simulink model "Simulatore_COMAU_UBHAND_IV_manipolazione_interna"  (Simulink 7.6)
settings: Solver=ode45  StartTime=0.0  StopTime=inf  RelTol=1e-3
values from neighbouring files (symbols this model uses):
  a_f = zeros(4,5)    [KinematicData.m]
  alpha_f = zeros(4,5)    [KinematicData.m]
  d_f = zeros(4,5)    [KinematicData.m]
  dc4_1 = 660    [KinematicData.m]
  T6HAND = [1 0 0 0;0 1 0 0; 0 0 1 357.48+95; 0 0 0 1]    [KinematicData.m]
  teta_d = atan(647.07/130)    [KinematicData.m]
  teta_f = zeros(4,5)    [KinematicData.m]
undefined symbols (not defined in the file or in its folder): a_c, a_w, alpha_c, alpha_w, d_c, d_w, Dt_fingers, teta_c
libraries: simulink x281, dspmtrx3 x171, aerolibutil x163, aerolibconvert2 x64, simulink_extras x46, vrlib x26, aerolibtransform2 x7, dspnetwork x1
(part 9 of 15)

== system Simulatore_COMAU_UBHAND_IV_manipolazione_interna/Work Space Control/Inverse_kinematic/Inverse_kinematic_comau/first solution/q1 ==
  P06 : Inport
  Divide : Product  Inputs=*/
  Gain : Gain  Gain=-1
  P06(1) : dspmtrx3/Submatrix  RowSpan=One row  RowStartMode=Index  RowStartIndex=1  RowEndMode=Index  RowEndIndex=3  ColSpan=One column  ColStartMode=Index  ColStartIndex=1  ColEndMode=Offset from last  ColEndIndex=1
  P06(2) : dspmtrx3/Submatrix  RowSpan=One row  RowStartMode=Index  RowStartIndex=2  RowEndMode=Index  RowEndIndex=3  ColSpan=One column  ColStartMode=Index  ColStartIndex=1  ColEndMode=Offset from last  ColEndIndex=1
  Trigonometric Function : Trigonometry  Operator=atan
  q1 : Outport
  wires:
  P06/1 -> P06(2)/1, P06(1)/1
  Trigonometric Function/1 -> Gain/1
  ? -> ?
  P06(2)/1 -> Divide/1
  P06(1)/1 -> Divide/2
  Divide/1 -> Trigonometric Function/1
  Gain/1 -> q1/1

== system Simulatore_COMAU_UBHAND_IV_manipolazione_interna/Work Space Control/Inverse_kinematic/Inverse_kinematic_comau/first solution/q2 ==
  P06 : Inport
  q1 : Inport  Port=2
  e_quadro : Inport  Port=3
  Add1 : Sum  Inputs=+-
  Add2 : Sum  Inputs=++-
  Add3 : Sum  Inputs=+-
  Add4 : Sum  Inputs=+-
  Angle Conversion1 : aerolibconvert2/Angle Conversion  IU=rad  OU=deg
  Angle Conversion2 : aerolibconvert2/Angle Conversion  IU=rad  OU=deg
  Constant1 : Constant  Value=2
  Constant2 : Constant  Value=a_c(2)
  Constant3 : Constant  Value=dc4_1
  Constant4 : Constant  Value=450
  Constant7 : Constant  Value=a_c(1)
  Display : Display
  Display1 : Display
  Display2 : Display
  Divide4 : Product  Inputs=***
  Divide5 : Product  Inputs=*/
  Math Function1 : Math  Operator=sqrt  SaturateOnIntegerOverflow=on
  Math Function5 : Math  Operator=square  SaturateOnIntegerOverflow=on
  Math Function6 : Math  Operator=square  SaturateOnIntegerOverflow=on
  P06(1) : dspmtrx3/Submatrix  RowSpan=One row  RowStartMode=Index  RowStartIndex=1  RowEndMode=Index  RowEndIndex=3  ColSpan=One column  ColStartMode=Index  ColStartIndex=1  ColEndMode=Offset from last  ColEndIndex=1
  P06(3) : dspmtrx3/Submatrix  RowSpan=One row  RowStartMode=Index  RowStartIndex=3  RowEndMode=Index  RowEndIndex=3  ColSpan=One column  ColStartMode=Index  ColStartIndex=1  ColEndMode=Offset from last  ColEndIndex=1
  Trigonometric Function2 : Trigonometry  Operator=cos
  Trigonometric Function3 : Trigonometry  Operator=acos
  cos_teta : Product  Inputs=*/
  fi : Trigonometry  Operator=atan2
  q2 : Outport
  wires:
  P06/1 -> P06(1)/1, P06(3)/1
  fi/1 -> Add3/1, Angle Conversion1/1
  Trigonometric Function3/1 -> Add3/2, Angle Conversion2/1
  Add3/1 -> q2/1
  q1/1 -> Trigonometric Function2/1
  P06(1)/1 -> Divide5/1
  Trigonometric Function2/1 -> Divide5/2
  Divide5/1 -> Add4/1
  Constant7/1 -> Add4/2
  Angle Conversion1/1 -> Display/1
  Angle Conversion2/1 -> Display1/1
  P06(3)/1 -> Add1/1
  Constant4/1 -> Add1/2
  Constant2/1 -> Divide4/2, Math Function5/1
  Constant3/1 -> Math Function6/1
  Constant1/1 -> Divide4/1
  e_quadro/1 -> Add2/1, Math Function1/1
  Math Function5/1 -> Add2/2
  Math Function6/1 -> Add2/3
  Math Function1/1 -> Divide4/3
  Divide4/1 -> cos_teta/2
  Add2/1 -> cos_teta/1
  cos_teta/1 -> Trigonometric Function3/1, Display2/1
  Add4/1 -> fi/1
  Add1/1 -> fi/2

== system Simulatore_COMAU_UBHAND_IV_manipolazione_interna/Work Space Control/Inverse_kinematic/Inverse_kinematic_hand/Inverse_kinematic_little/Direct_kinematic_index ==
  Joints_reference : Inport  PortDimensions=3
  Constant : Constant  Value=[alpha_f(5,1) alpha_f(5,2) alpha_f(5,3) alpha_f(5,4)]
  Constant2 : Constant  Value=[a_f(5,1) a_f(5,2) a_f(5,3) a_f(5,4)]
  Degrees to Radians : simulink_extras/Transformations/Degrees to Radians
  Demux : Demux
  Demux1 : Demux
  Demux2 : Demux
  Demux3 : Demux  Outputs=3
  Display3 : Display
  Gain : Gain  Gain=0.7
  Matrix Multiply : Product  Multiplication=Matrix(*)
  Matrix Multiply1 : Product  Multiplication=Matrix(*)
  Matrix Multiply2 : Product  Multiplication=Matrix(*)
  Subsystem : Subsystem
  Subsystem1 : Subsystem
  Subsystem2 : Subsystem
  Subsystem3 : Subsystem
  Vector Concatenate : Concatenate  Mode=Vector
  T_finger_tip : Outport
  T_01 : Outport  Port=2
  T_02 : Outport  Port=3
  T_03 : Outport  Port=4
  wires:
  Degrees to Radians/1 -> Demux/1
  Vector Concatenate/1 -> Display3/1, Degrees to Radians/1
  Gain/1 -> Vector Concatenate/2
  Demux3/3 -> Gain/1
  Joints_reference/1 -> Vector Concatenate/1, Demux3/1
  Demux1/4 -> Subsystem3/2(alpha)
  Demux1/3 -> Subsystem2/2(alpha)
  Demux1/2 -> Subsystem1/2(alpha)
  Demux1/1 -> Subsystem/2(alpha)
  Demux2/4 -> Subsystem3/3(a)
  Demux2/3 -> Subsystem2/3(a)
  Demux2/2 -> Subsystem1/3(a)
  Demux2/1 -> Subsystem/3(a)
  Demux/4 -> Subsystem3/1(q)
  Demux/3 -> Subsystem2/1(q)
  Demux/2 -> Subsystem1/1(q)
  Demux/1 -> Subsystem/1(q)
  Constant2/1 -> Demux2/1
  Matrix Multiply2/1 -> T_finger_tip/1, T_03/1
  Matrix Multiply1/1 -> Matrix Multiply2/1
  Subsystem3/1(Out1) -> Matrix Multiply2/2
  Subsystem2/1(Out1) -> Matrix Multiply1/2
  Matrix Multiply/1 -> Matrix Multiply1/1, T_02/1
  Subsystem1/1(Out1) -> Matrix Multiply/2
  Constant/1 -> Demux1/1
  Subsystem/1(Out1) -> Matrix Multiply/1, T_01/1

== system Simulatore_COMAU_UBHAND_IV_manipolazione_interna/Work Space Control/Inverse_kinematic/Inverse_kinematic_hand/Inverse_kinematic_little/jacobian ==
  T_01 : Inport
  T_02 : Inport  Port=2
  T_03 : Inport  Port=3
  T_base : Inport  Port=4
  Display1 : Display
  Matrix Concatenate3 : Concatenate  NumInputs=3  Mode=Multidimensional array  ConcatenateDimension=2
  Matrix Concatenate4 : Concatenate  NumInputs=3  Mode=Multidimensional array  ConcatenateDimension=2
  Matrix Concatenate5 : Concatenate  Mode=Multidimensional array
  z1 : Subsystem
  J : Outport
  wires:
  z1/6(z2xpe-p2) -> Matrix Concatenate4/3
  z1/1(z0) -> Matrix Concatenate3/1
  z1/2(z1) -> Matrix Concatenate3/2
  z1/3(z2) -> Matrix Concatenate3/3
  z1/4(z0xpe-p0) -> Matrix Concatenate4/1
  z1/5(z1xpe-p1) -> Matrix Concatenate4/2
  Matrix Concatenate4/1 -> J/1
  T_01/1 -> z1/1(T_01)
  T_02/1 -> z1/2(T_02)
  T_03/1 -> z1/3(T_03)
  T_base/1 -> z1/4(T_base)

== system Simulatore_COMAU_UBHAND_IV_manipolazione_interna/Work Space Control/Inverse_kinematic/Inverse_kinematic_hand/Inverse_kinematic_middle/Direct_kinematic_index ==
  Joints_reference : Inport  PortDimensions=3
  Constant : Constant  Value=[alpha_f(3,1) alpha_f(3,2) alpha_f(3,3) alpha_f(3,4)]
  Constant2 : Constant  Value=[a_f(3,1) a_f(3,2) a_f(3,3) a_f(3,4)]
  Degrees to Radians : simulink_extras/Transformations/Degrees to Radians
  Demux : Demux
  Demux1 : Demux
  Demux2 : Demux
  Demux3 : Demux  Outputs=3
  Display3 : Display
  Gain : Gain  Gain=0.7
  Matrix Multiply : Product  Multiplication=Matrix(*)
  Matrix Multiply1 : Product  Multiplication=Matrix(*)
  Matrix Multiply2 : Product  Multiplication=Matrix(*)
  Subsystem : Subsystem
  Subsystem1 : Subsystem
  Subsystem2 : Subsystem
  Subsystem3 : Subsystem
  Vector Concatenate : Concatenate  Mode=Vector
  T_finger_tip : Outport
  T_01 : Outport  Port=2
  T_02 : Outport  Port=3
  T_03 : Outport  Port=4
  wires:
  Degrees to Radians/1 -> Demux/1
  Vector Concatenate/1 -> Display3/1, Degrees to Radians/1
  Gain/1 -> Vector Concatenate/2
  Demux3/3 -> Gain/1
  Joints_reference/1 -> Vector Concatenate/1, Demux3/1
  Demux1/4 -> Subsystem3/2(alpha)
  Demux1/3 -> Subsystem2/2(alpha)
  Demux1/2 -> Subsystem1/2(alpha)
  Demux1/1 -> Subsystem/2(alpha)
  Demux2/4 -> Subsystem3/3(a)
  Demux2/3 -> Subsystem2/3(a)
  Demux2/2 -> Subsystem1/3(a)
  Demux2/1 -> Subsystem/3(a)
  Demux/4 -> Subsystem3/1(q)
  Demux/3 -> Subsystem2/1(q)
  Demux/2 -> Subsystem1/1(q)
  Demux/1 -> Subsystem/1(q)
  Constant2/1 -> Demux2/1
  Matrix Multiply2/1 -> T_finger_tip/1, T_03/1
  Matrix Multiply1/1 -> Matrix Multiply2/1
  Subsystem3/1(Out1) -> Matrix Multiply2/2
  Subsystem2/1(Out1) -> Matrix Multiply1/2
  Matrix Multiply/1 -> Matrix Multiply1/1, T_02/1
  Subsystem1/1(Out1) -> Matrix Multiply/2
  Constant/1 -> Demux1/1
  Subsystem/1(Out1) -> Matrix Multiply/1, T_01/1

== system Simulatore_COMAU_UBHAND_IV_manipolazione_interna/Work Space Control/Inverse_kinematic/Inverse_kinematic_hand/Inverse_kinematic_middle/jacobian ==
  T_01 : Inport
  T_02 : Inport  Port=2
  T_03 : Inport  Port=3
  T_base : Inport  Port=4
  Display1 : Display
  Matrix Concatenate3 : Concatenate  NumInputs=3  Mode=Multidimensional array  ConcatenateDimension=2
  Matrix Concatenate4 : Concatenate  NumInputs=3  Mode=Multidimensional array  ConcatenateDimension=2
  Matrix Concatenate5 : Concatenate  Mode=Multidimensional array
  z1 : Subsystem
  J : Outport
  wires:
  T_01/1 -> z1/1(T_01)
  T_02/1 -> z1/2(T_02)
  T_03/1 -> z1/3(T_03)
  z1/6(z2xpe-p2) -> Matrix Concatenate4/3
  z1/1(z0) -> Matrix Concatenate3/1
  z1/2(z1) -> Matrix Concatenate3/2
  z1/3(z2) -> Matrix Concatenate3/3
  z1/4(z0xpe-p0) -> Matrix Concatenate4/1
  z1/5(z1xpe-p1) -> Matrix Concatenate4/2
  T_base/1 -> z1/4(T_base)
  Matrix Concatenate4/1 -> J/1

== system Simulatore_COMAU_UBHAND_IV_manipolazione_interna/Work Space Control/Inverse_kinematic/Inverse_kinematic_hand/Inverse_kinematic_middle1/Direct_kinematic_index ==
  Joints_reference : Inport  PortDimensions=3
  Constant : Constant  Value=[alpha_f(2,1) alpha_f(2,2) alpha_f(2,3) alpha_f(2,4)]
  Constant2 : Constant  Value=[a_f(2,1) a_f(2,2) a_f(2,3) a_f(2,4)]
  Degrees to Radians : simulink_extras/Transformations/Degrees to Radians
  Demux : Demux
  Demux1 : Demux
  Demux2 : Demux
  Demux3 : Demux  Outputs=3
  Display3 : Display
  Gain : Gain  Gain=0.7
  Matrix Multiply : Product  Multiplication=Matrix(*)
  Matrix Multiply1 : Product  Multiplication=Matrix(*)
  Matrix Multiply2 : Product  Multiplication=Matrix(*)
  Subsystem : Subsystem
  Subsystem1 : Subsystem
  Subsystem2 : Subsystem
  Subsystem3 : Subsystem
  Vector Concatenate : Concatenate  Mode=Vector
  T_finger_tip : Outport
  T_01 : Outport  Port=2
  T_02 : Outport  Port=3
  T_03 : Outport  Port=4
  wires:
  Degrees to Radians/1 -> Demux/1
  Vector Concatenate/1 -> Display3/1, Degrees to Radians/1
  Gain/1 -> Vector Concatenate/2
  Demux3/3 -> Gain/1
  Joints_reference/1 -> Vector Concatenate/1, Demux3/1
  Demux1/4 -> Subsystem3/2(alpha)
  Demux1/3 -> Subsystem2/2(alpha)
  Demux1/2 -> Subsystem1/2(alpha)
  Demux1/1 -> Subsystem/2(alpha)
  Demux2/4 -> Subsystem3/3(a)
  Demux2/3 -> Subsystem2/3(a)
  Demux2/2 -> Subsystem1/3(a)
  Demux2/1 -> Subsystem/3(a)
  Demux/4 -> Subsystem3/1(q)
  Demux/3 -> Subsystem2/1(q)
  Demux/2 -> Subsystem1/1(q)
  Demux/1 -> Subsystem/1(q)
  Constant2/1 -> Demux2/1
  Matrix Multiply2/1 -> T_finger_tip/1, T_03/1
  Matrix Multiply1/1 -> Matrix Multiply2/1
  Subsystem3/1(Out1) -> Matrix Multiply2/2
  Subsystem2/1(Out1) -> Matrix Multiply1/2
  Matrix Multiply/1 -> Matrix Multiply1/1, T_02/1
  Subsystem1/1(Out1) -> Matrix Multiply/2
  Constant/1 -> Demux1/1
  Subsystem/1(Out1) -> Matrix Multiply/1, T_01/1

== system Simulatore_COMAU_UBHAND_IV_manipolazione_interna/Work Space Control/Inverse_kinematic/Inverse_kinematic_hand/Inverse_kinematic_middle1/jacobian ==
  T_01 : Inport
  T_02 : Inport  Port=2
  T_03 : Inport  Port=3
  T_base : Inport  Port=4
  Matrix Concatenate3 : Concatenate  NumInputs=3  Mode=Multidimensional array  ConcatenateDimension=2
  Matrix Concatenate4 : Concatenate  NumInputs=3  Mode=Multidimensional array  ConcatenateDimension=2
  z1 : Subsystem
  J : Outport
  wires:
  T_01/1 -> z1/1(T_01)
  T_02/1 -> z1/2(T_02)
  T_03/1 -> z1/3(T_03)
  z1/6(z2xpe-p2) -> Matrix Concatenate4/3
  z1/1(z0) -> Matrix Concatenate3/1
  z1/2(z1) -> Matrix Concatenate3/2
  z1/3(z2) -> Matrix Concatenate3/3
  z1/4(z0xpe-p0) -> Matrix Concatenate4/1
  z1/5(z1xpe-p1) -> Matrix Concatenate4/2
  T_base/1 -> z1/4(T_base)
  Matrix Concatenate4/1 -> J/1

== system Simulatore_COMAU_UBHAND_IV_manipolazione_interna/Work Space Control/Inverse_kinematic/Inverse_kinematic_hand/Inverse_kinematic_ring/Direct_kinematic_index ==
  Joints_reference : Inport  PortDimensions=3
  Constant : Constant  Value=[alpha_f(4,1) alpha_f(4,2) alpha_f(4,3) alpha_f(4,4)]
  Constant2 : Constant  Value=[a_f(4,1) a_f(4,2) a_f(4,3) a_f(4,4)]
  Degrees to Radians : simulink_extras/Transformations/Degrees to Radians
  Demux : Demux
  Demux1 : Demux
  Demux2 : Demux
  Demux3 : Demux  Outputs=3
  Display3 : Display
  Gain : Gain  Gain=0.7
  Matrix Multiply : Product  Multiplication=Matrix(*)
  Matrix Multiply1 : Product  Multiplication=Matrix(*)
  Matrix Multiply2 : Product  Multiplication=Matrix(*)
  Subsystem : Subsystem
  Subsystem1 : Subsystem
  Subsystem2 : Subsystem
  Subsystem3 : Subsystem
  Vector Concatenate : Concatenate  Mode=Vector
  T_finger_tip : Outport
  T_01 : Outport  Port=2
  T_02 : Outport  Port=3
  T_03 : Outport  Port=4
  wires:
  Degrees to Radians/1 -> Demux/1
  Vector Concatenate/1 -> Display3/1, Degrees to Radians/1
  Gain/1 -> Vector Concatenate/2
  Demux3/3 -> Gain/1
  Joints_reference/1 -> Vector Concatenate/1, Demux3/1
  Demux1/4 -> Subsystem3/2(alpha)
  Demux1/3 -> Subsystem2/2(alpha)
  Demux1/2 -> Subsystem1/2(alpha)
  Demux1/1 -> Subsystem/2(alpha)
  Demux2/4 -> Subsystem3/3(a)
  Demux2/3 -> Subsystem2/3(a)
  Demux2/2 -> Subsystem1/3(a)
  Demux2/1 -> Subsystem/3(a)
  Demux/4 -> Subsystem3/1(q)
  Demux/3 -> Subsystem2/1(q)
  Demux/2 -> Subsystem1/1(q)
  Demux/1 -> Subsystem/1(q)
  Constant2/1 -> Demux2/1
  Matrix Multiply2/1 -> T_finger_tip/1, T_03/1
  Matrix Multiply1/1 -> Matrix Multiply2/1
  Subsystem3/1(Out1) -> Matrix Multiply2/2
  Subsystem2/1(Out1) -> Matrix Multiply1/2
  Matrix Multiply/1 -> Matrix Multiply1/1, T_02/1
  Subsystem1/1(Out1) -> Matrix Multiply/2
  Constant/1 -> Demux1/1
  Subsystem/1(Out1) -> Matrix Multiply/1, T_01/1

== system Simulatore_COMAU_UBHAND_IV_manipolazione_interna/Work Space Control/Inverse_kinematic/Inverse_kinematic_hand/Inverse_kinematic_ring/jacobian ==
  T_01 : Inport
  T_02 : Inport  Port=2
  T_03 : Inport  Port=3
  T_base : Inport  Port=4
  Display1 : Display
  Matrix Concatenate3 : Concatenate  NumInputs=3  Mode=Multidimensional array  ConcatenateDimension=2
  Matrix Concatenate4 : Concatenate  NumInputs=3  Mode=Multidimensional array  ConcatenateDimension=2
  Matrix Concatenate5 : Concatenate  Mode=Multidimensional array
  z1 : Subsystem
  J : Outport
  wires:
  T_base/1 -> z1/4(T_base)
  z1/6(z2xpe-p2) -> Matrix Concatenate4/3
  z1/1(z0) -> Matrix Concatenate3/1
  z1/2(z1) -> Matrix Concatenate3/2
  z1/3(z2) -> Matrix Concatenate3/3
  z1/4(z0xpe-p0) -> Matrix Concatenate4/1
  z1/5(z1xpe-p1) -> Matrix Concatenate4/2
  Matrix Concatenate4/1 -> J/1
  T_03/1 -> z1/3(T_03)
  T_02/1 -> z1/2(T_02)
  T_01/1 -> z1/1(T_01)

== system Simulatore_COMAU_UBHAND_IV_manipolazione_interna/Work Space Control/Inverse_kinematic/Inverse_kinematic_hand/Inverse_kinematic_thumb/Direct_kinematic_thumb ==
  Joints_reference : Inport  PortDimensions=3
  Add{,1..4} (5 alike) : Sum
  Constant : Constant  Value=[alpha_f(1,1) alpha_f(1,2) alpha_f(1,3) alpha_f(1,4)]
  Constant1 : Constant  Value=teta_f(1,1)
  Constant2 : Constant  Value=[a_f(1,1) a_f(1,2) a_f(1,3) a_f(1,4)]
  Constant3 : Constant  Value=teta_f(1,2)
  Constant4 : Constant  Value=teta_f(1,3)
  Constant5 : Constant  Value=teta_f(1,4)
  Constant6 : Constant  Value=-20
  Degrees to Radians : simulink_extras/Transformations/Degrees to Radians
  Demux : Demux
  Demux1 : Demux
  Demux2 : Demux
  Demux3 : Demux  Outputs=3
  Display3 : Display
  Gain : Gain  Gain=0.8
  Matrix Multiply : Product  Multiplication=Matrix(*)
  Matrix Multiply1 : Product  Multiplication=Matrix(*)
  Matrix Multiply2 : Product  Multiplication=Matrix(*)
  Subsystem : Subsystem
  Subsystem1 : Subsystem
  Subsystem2 : Subsystem
  Subsystem3 : Subsystem
  Vector Concatenate : Concatenate  Mode=Vector
  T_finger_tip : Outport
  T_01 : Outport  Port=2
  T_02 : Outport  Port=3
  T_03 : Outport  Port=4
  wires:
  Constant/1 -> Demux1/1
  Subsystem1/1(Out1) -> Matrix Multiply/2
  Matrix Multiply/1 -> Matrix Multiply1/1, T_02/1
  Subsystem2/1(Out1) -> Matrix Multiply1/2
  Subsystem3/1(Out1) -> Matrix Multiply2/2
  Matrix Multiply1/1 -> Matrix Multiply2/1
  Matrix Multiply2/1 -> T_finger_tip/1, T_03/1
  Constant2/1 -> Demux2/1
  Demux2/1 -> Subsystem/3(a)
  Demux2/2 -> Subsystem1/3(a)
  Demux2/3 -> Subsystem2/3(a)
  Demux2/4 -> Subsystem3/3(a)
  Demux1/1 -> Subsystem/2(alpha)
  Demux1/2 -> Subsystem1/2(alpha)
  Demux1/3 -> Subsystem2/2(alpha)
  Demux1/4 -> Subsystem3/2(alpha)
  Joints_reference/1 -> Demux3/1, Vector Concatenate/1
  Demux3/3 -> Gain/1
  Vector Concatenate/1 -> Display3/1, Degrees to Radians/1
  Degrees to Radians/1 -> Demux/1
  Constant1/1 -> Add/2
  Demux/1 -> Add/1
  Demux/2 -> Add1/1
  Demux/3 -> Add2/1
  Demux/4 -> Add3/1
  Add2/1 -> Subsystem2/1(q)
  Add1/1 -> Subsystem1/1(q)
  Add/1 -> Subsystem/1(q)
  Constant3/1 -> Add1/2
  Constant4/1 -> Add2/2
  Constant5/1 -> Add3/2
  Constant6/1 -> Add4/2
  Gain/1 -> Add4/1
  Add4/1 -> Vector Concatenate/2
  Add3/1 -> Subsystem3/1(q)
  Subsystem/1(Out1) -> Matrix Multiply/1, T_01/1

== system Simulatore_COMAU_UBHAND_IV_manipolazione_interna/Work Space Control/Inverse_kinematic/Inverse_kinematic_hand/Inverse_kinematic_thumb/jacobian ==
  T_01 : Inport
  T_02 : Inport  Port=2
  T_03 : Inport  Port=3
  T_base : Inport  Port=4
  Display1 : Display
  Matrix Concatenate3 : Concatenate  NumInputs=3  Mode=Multidimensional array  ConcatenateDimension=2
  Matrix Concatenate4 : Concatenate  NumInputs=3  Mode=Multidimensional array  ConcatenateDimension=2
  Matrix Concatenate5 : Concatenate  Mode=Multidimensional array
  z1 : Subsystem
  J : Outport
  wires:
  T_01/1 -> z1/1(T_01)
  T_02/1 -> z1/2(T_02)
  T_03/1 -> z1/3(T_03)
  T_base/1 -> z1/4(T_base)
  z1/6(z2xpe-p2) -> Matrix Concatenate4/3
  z1/1(z0) -> Matrix Concatenate3/1
  z1/2(z1) -> Matrix Concatenate3/2
  z1/3(z2) -> Matrix Concatenate3/3
  z1/4(z0xpe-p0) -> Matrix Concatenate4/1
  z1/5(z1xpe-p1) -> Matrix Concatenate4/2
  Matrix Concatenate4/1 -> J/1
  Matrix Concatenate3/1 -> Display1/1

== system Simulatore_COMAU_UBHAND_IV_manipolazione_interna/Work Space Control/Inverse_kinematic/Tf0/T_0_8/Subsystem ==
  q : Inport
  alpha : Inport  Port=2
  a : Inport  Port=3
  A_01 : aerolibutil/Create 3x3 Matrix
  Constant : Constant  Value=0
  Constant1 : Constant  Value=[0 0 0 1]  VectorParams1D=off
  Constant5 : Constant  Value=d_w(1)
  Display : Display
  Gain : Gain  Gain=-1
  Gain1 : Gain  Gain=-1
  Matrix Concatenation : Concatenate  Mode=Multidimensional array  ConcatenateDimension=2
  Matrix Concatenation1 : Concatenate  Mode=Multidimensional array
  Product{,1..5} (6 alike) : Product  RndMeth=Zero
  Trigonometric Function{,1,6..8,11..12} (7 alike) : Trigonometry  Operator=cos
  Trigonometric Function{2..5,9..10,13} (7 alike) : Trigonometry
  Vector Concatenate : Concatenate  NumInputs=3  Mode=Multidimensional array
  Out1 : Outport
  wires:
  Matrix Concatenation1/1 -> Display/1, Out1/1
  Constant1/1 -> Matrix Concatenation1/2
  Matrix Concatenation/1 -> Matrix Concatenation1/1
  Vector Concatenate/1 -> Matrix Concatenation/2
  A_01/1 -> Matrix Concatenation/1
  Constant5/1 -> Vector Concatenate/3
  Product5/1 -> Vector Concatenate/2
  Product4/1 -> Vector Concatenate/1
  Trigonometric Function13/1 -> Product5/2
  Trigonometric Function12/1 -> Product4/2
  a/1 -> Product5/1, Product4/1
  Trigonometric Function11/1 -> A_01/9
  Trigonometric Function10/1 -> A_01/8
  Constant/1 -> A_01/7
  Gain1/1 -> A_01/6
  Product2/1 -> A_01/5
  Trigonometric Function5/1 -> A_01/4
  Product1/1 -> A_01/3
  Gain/1 -> A_01/2
  Trigonometric Function/1 -> A_01/1
  Product/1 -> Gain/1
  Product3/1 -> Gain1/1
  Trigonometric Function9/1 -> Product3/2
  Trigonometric Function8/1 -> Product3/1
  Trigonometric Function7/1 -> Product2/2
  Trigonometric Function6/1 -> Product2/1
  Trigonometric Function4/1 -> Product1/2
  Trigonometric Function3/1 -> Product1/1
  alpha/1 -> Trigonometric Function11/1, Trigonometric Function10/1, Trigonometric Function9/1, Trigonometric Function7/1, Trigonometric Function4/1, Trigonometric Function1/1
  q/1 -> Trigonometric Function13/1, Trigonometric Function12/1, Trigonometric Function8/1, Trigonometric Function6/1, Trigonometric Function5/1, Trigonometric Function3/1, Trigonometric Function2/1, Trigonometric Function/1
  Trigonometric Function1/1 -> Product/2
  Trigonometric Function2/1 -> Product/1

== system Simulatore_COMAU_UBHAND_IV_manipolazione_interna/Work Space Control/Inverse_kinematic/Tf0/T_0_8/Subsystem1 ==
  q : Inport
  alpha : Inport  Port=2
  a : Inport  Port=3
  A_01 : aerolibutil/Create 3x3 Matrix
  Constant : Constant  Value=0
  Constant1 : Constant  Value=[0 0 0 1]  VectorParams1D=off
  Constant5 : Constant  Value=d_w(2)
  Display : Display
  Gain : Gain  Gain=-1
  Gain1 : Gain  Gain=-1
  Matrix Concatenation : Concatenate  Mode=Multidimensional array  ConcatenateDimension=2
  Matrix Concatenation1 : Concatenate  Mode=Multidimensional array
  Product{,1..5} (6 alike) : Product  RndMeth=Zero
  Trigonometric Function{,1,6..8,11..12} (7 alike) : Trigonometry  Operator=cos
  Trigonometric Function{2..5,9..10,13} (7 alike) : Trigonometry
  Vector Concatenate : Concatenate  NumInputs=3  Mode=Multidimensional array
  Out1 : Outport
  wires:
  Trigonometric Function2/1 -> Product/1
  Trigonometric Function1/1 -> Product/2
  q/1 -> Trigonometric Function/1, Trigonometric Function2/1, Trigonometric Function3/1, Trigonometric Function5/1, Trigonometric Function6/1, Trigonometric Function8/1, Trigonometric Function12/1, Trigonometric Function13/1
  alpha/1 -> Trigonometric Function1/1, Trigonometric Function4/1, Trigonometric Function7/1, Trigonometric Function9/1, Trigonometric Function10/1, Trigonometric Function11/1
  Trigonometric Function3/1 -> Product1/1
  Trigonometric Function4/1 -> Product1/2
  Trigonometric Function6/1 -> Product2/1
  Trigonometric Function7/1 -> Product2/2
  Trigonometric Function8/1 -> Product3/1
  Trigonometric Function9/1 -> Product3/2
  Product3/1 -> Gain1/1
  Product/1 -> Gain/1
  Trigonometric Function/1 -> A_01/1
  Gain/1 -> A_01/2
  Product1/1 -> A_01/3
  Trigonometric Function5/1 -> A_01/4
  Product2/1 -> A_01/5
  Gain1/1 -> A_01/6
  Constant/1 -> A_01/7
  Trigonometric Function10/1 -> A_01/8
  Trigonometric Function11/1 -> A_01/9
  a/1 -> Product5/1, Product4/1
  Trigonometric Function12/1 -> Product4/2
  Trigonometric Function13/1 -> Product5/2
  Product4/1 -> Vector Concatenate/1
  Product5/1 -> Vector Concatenate/2
  Constant5/1 -> Vector Concatenate/3
  A_01/1 -> Matrix Concatenation/1
  Vector Concatenate/1 -> Matrix Concatenation/2
  Matrix Concatenation/1 -> Matrix Concatenation1/1
  Constant1/1 -> Matrix Concatenation1/2
  Matrix Concatenation1/1 -> Out1/1, Display/1

== system Simulatore_COMAU_UBHAND_IV_manipolazione_interna/Work Space Control/Inverse_kinematic/Tf0/T_0_8/wrist_reference_ ==
  Angle Conversion : aerolibconvert2/Angle Conversion  IU=deg  OU=rad
  Constant1 : Constant  Value=[0 90 0]  VectorParams1D=off
  Constant2 : Constant  Value=[0 0 0 ]'  VectorParams1D=off
  Constant4 : Constant  Value=[0 0 0 1]  VectorParams1D=off
  Matrix Concatenate : Concatenate  Mode=Multidimensional array  ConcatenateDimension=2
  Matrix Concatenate1 : Concatenate  Mode=Multidimensional array
  Rotation Angles to Direction Cosine Matrix : aerolibtransform2/Rotation Angles to Direction Cosine Matrix  rotationOrder=ZYX
  TH_W : Outport
  wires:
  Matrix Concatenate1/1 -> TH_W/1
  Rotation Angles to Direction Cosine Matrix/1 -> Matrix Concatenate/1
  Constant4/1 -> Matrix Concatenate1/2
  Matrix Concatenate/1 -> Matrix Concatenate1/1
  Angle Conversion/1 -> Rotation Angles to Direction Cosine Matrix/1
  Constant1/1 -> Angle Conversion/1
  Constant2/1 -> Matrix Concatenate/2

== system Simulatore_COMAU_UBHAND_IV_manipolazione_interna/Work Space Control/Grasp Planner/SCOPES/Direct_kinematic2/Direct_kinematic_Comau/T_0_3 ==
  q[1..3] : Inport
  Add : Sum
  Add1 : Sum
  Add2 : Sum  Inputs=-+
  Constant : Constant  Value=[alpha_c(1) alpha_c(2) alpha_c(3) ]
  Constant1 : Constant  Value=teta_c(2)
  Constant2 : Constant  Value=[a_c(1) a_c(2) a_c(3)]
  Constant3 : Constant  Value=teta_c(3)
  Constant4 : Constant  Value=teta_c(1)
  Degrees to Radians : simulink_extras/Transformations/Degrees to Radians
  Demux : Demux  Outputs=3
  Demux1 : Demux  Outputs=3
  Demux2 : Demux  Outputs=3
  Display3 : Display
  Matrix Multiply : Product  Multiplication=Matrix(*)
  Matrix Multiply1 : Product  Multiplication=Matrix(*)
  Subsystem : Subsystem
  Subsystem1 : Subsystem
  Subsystem2 : Subsystem
  T_0_3 : Outport
  wires:
  Constant/1 -> Demux1/1
  Subsystem/1(Out1) -> Matrix Multiply/1
  Subsystem1/1(Out1) -> Matrix Multiply/2
  Matrix Multiply/1 -> Matrix Multiply1/1
  Subsystem2/1(Out1) -> Matrix Multiply1/2
  Matrix Multiply1/1 -> T_0_3/1
  Constant2/1 -> Demux2/1
  Demux2/1 -> Subsystem/3(a)
  Demux2/2 -> Subsystem1/3(a)
  Demux2/3 -> Subsystem2/3(a)
  Demux1/1 -> Subsystem/2(alpha)
  Demux1/2 -> Subsystem1/2(alpha)
  Demux1/3 -> Subsystem2/2(alpha)
  Degrees to Radians/1 -> Demux/1
  q[1..3]/1 -> Degrees to Radians/1, Display3/1
  Add/1 -> Subsystem1/1(q)
  Constant1/1 -> Add/2
  Demux/2 -> Add/1
  Constant3/1 -> Add1/2
  Demux/3 -> Add1/1
  Add1/1 -> Subsystem2/1(q)
  Demux/1 -> Add2/1
  Add2/1 -> Subsystem/1(q)
  Constant4/1 -> Add2/2

== system Simulatore_COMAU_UBHAND_IV_manipolazione_interna/Work Space Control/Grasp Planner/SCOPES/Direct_kinematic2/Direct_kinematic_Comau/T_3_6 ==
  q[1..3] : Inport
  Add : Sum  Inputs=-+
  Add1 : Sum  Inputs=-+
  Add2 : Sum  Inputs=-+
  Constant : Constant  Value=[alpha_c(4) alpha_c(5) alpha_c(6) ]
  Constant1 : Constant  Value=teta_c(5)
  Constant2 : Constant  Value=[a_c(4) a_c(5) a_c(6)]
  Constant3 : Constant  Value=teta_c(6)
  Constant4 : Constant  Value=teta_c(4)
  Degrees to Radians : simulink_extras/Transformations/Degrees to Radians
  Demux : Demux  Outputs=3
  Demux1 : Demux  Outputs=3
  Demux2 : Demux  Outputs=3
  Display3 : Display
  Matrix Multiply : Product  Multiplication=Matrix(*)
  Matrix Multiply1 : Product  Multiplication=Matrix(*)
  Subsystem : Subsystem
  Subsystem1 : Subsystem
  Subsystem2 : Subsystem
  T_3_6 : Outport
  wires:
  q[1..3]/1 -> Display3/1, Degrees to Radians/1
  Degrees to Radians/1 -> Demux/1
  Demux1/3 -> Subsystem2/2(alpha)
  Demux1/2 -> Subsystem1/2(alpha)
  Demux1/1 -> Subsystem/2(alpha)
  Demux2/3 -> Subsystem2/3(a)
  Demux2/2 -> Subsystem1/3(a)
  Demux2/1 -> Subsystem/3(a)
  Constant2/1 -> Demux2/1
  Matrix Multiply1/1 -> T_3_6/1
  Subsystem2/1(Out1) -> Matrix Multiply1/2
  Matrix Multiply/1 -> Matrix Multiply1/1
  Subsystem1/1(Out1) -> Matrix Multiply/2
  Subsystem/1(Out1) -> Matrix Multiply/1
  Constant/1 -> Demux1/1
  Add/1 -> Subsystem1/1(q)
  Constant1/1 -> Add/2
  Demux/2 -> Add/1
  Constant3/1 -> Add1/2
  Demux/3 -> Add1/1
  Demux/1 -> Add2/1
  Add2/1 -> Subsystem/1(q)
  Constant4/1 -> Add2/2
  Add1/1 -> Subsystem2/1(q)

== system Simulatore_COMAU_UBHAND_IV_manipolazione_interna/Work Space Control/Grasp Planner/SCOPES/Direct_kinematic2/Direct_kinematic_hand/Direct_kinematic_index ==
  Joints_reference : Inport  PortDimensions=3
  T_0f : Inport  Port=2
  Constant : Constant  Value=[alpha_f(2,1) alpha_f(2,2) alpha_f(2,3) alpha_f(2,4)]
  Constant2 : Constant  Value=[a_f(2,1) a_f(2,2) a_f(2,3) a_f(2,4)]
  Degrees to Radians : simulink_extras/Transformations/Degrees to Radians
  Demux : Demux
  Demux1 : Demux
  Demux2 : Demux
  Demux3 : Demux  Outputs=3
  Display3 : Display
  Gain : Gain  Gain=0.7
  Matrix Multiply{,1..3} (4 alike) : Product  Multiplication=Matrix(*)
  Subsystem : Subsystem
  Subsystem1 : Subsystem
  Subsystem2 : Subsystem
  Subsystem3 : Subsystem
  Vector Concatenate : Concatenate  Mode=Vector
  T_finger_tip : Outport
  wires:
  Degrees to Radians/1 -> Demux/1
  Vector Concatenate/1 -> Display3/1, Degrees to Radians/1
  Gain/1 -> Vector Concatenate/2
  Demux3/3 -> Gain/1
  Joints_reference/1 -> Vector Concatenate/1, Demux3/1
  Demux1/4 -> Subsystem3/2(alpha)
  Demux1/3 -> Subsystem2/2(alpha)
  Demux1/2 -> Subsystem1/2(alpha)
  Demux1/1 -> Subsystem/2(alpha)
  Demux2/4 -> Subsystem3/3(a)
  Demux2/3 -> Subsystem2/3(a)
  Demux2/2 -> Subsystem1/3(a)
  Demux2/1 -> Subsystem/3(a)
  Demux/4 -> Subsystem3/1(q)
  Demux/3 -> Subsystem2/1(q)
  Demux/2 -> Subsystem1/1(q)
  Demux/1 -> Subsystem/1(q)
  Constant2/1 -> Demux2/1
  Matrix Multiply2/1 -> T_finger_tip/1
  Matrix Multiply1/1 -> Matrix Multiply2/1
  Subsystem3/1(Out1) -> Matrix Multiply2/2
  Subsystem2/1(Out1) -> Matrix Multiply1/2
  Matrix Multiply/1 -> Matrix Multiply1/1
  Subsystem1/1(Out1) -> Matrix Multiply/2
  Subsystem/1(Out1) -> Matrix Multiply3/2
  Constant/1 -> Demux1/1
  T_0f/1 -> Matrix Multiply3/1
  Matrix Multiply3/1 -> Matrix Multiply/1

== system Simulatore_COMAU_UBHAND_IV_manipolazione_interna/Work Space Control/Grasp Planner/SCOPES/Direct_kinematic2/Direct_kinematic_hand/Direct_kinematic_little ==
  Joints_reference : Inport  PortDimensions=3
  T_0f : Inport  Port=2
  Constant : Constant  Value=[alpha_f(5,1) alpha_f(5,2) alpha_f(5,3) alpha_f(5,4)]
  Constant2 : Constant  Value=[a_f(5,1) a_f(5,2) a_f(5,3) a_f(5,4)]
  Degrees to Radians : simulink_extras/Transformations/Degrees to Radians
  Demux : Demux
  Demux1 : Demux
  Demux2 : Demux
  Demux3 : Demux  Outputs=3
  Display3 : Display
  Gain : Gain  Gain=0.7
  Matrix Multiply{,1..3} (4 alike) : Product  Multiplication=Matrix(*)
  Subsystem : Subsystem
  Subsystem1 : Subsystem
  Subsystem2 : Subsystem
  Subsystem3 : Subsystem
  Vector Concatenate : Concatenate  Mode=Vector
  T_finger_tip : Outport
  wires:
  Degrees to Radians/1 -> Demux/1
  Vector Concatenate/1 -> Display3/1, Degrees to Radians/1
  Gain/1 -> Vector Concatenate/2
  Demux3/3 -> Gain/1
  Joints_reference/1 -> Vector Concatenate/1, Demux3/1
  Demux1/4 -> Subsystem3/2(alpha)
  Demux1/3 -> Subsystem2/2(alpha)
  Demux1/2 -> Subsystem1/2(alpha)
  Demux1/1 -> Subsystem/2(alpha)
  Demux2/4 -> Subsystem3/3(a)
  Demux2/3 -> Subsystem2/3(a)
  Demux2/2 -> Subsystem1/3(a)
  Demux2/1 -> Subsystem/3(a)
  Demux/4 -> Subsystem3/1(q)
  Demux/3 -> Subsystem2/1(q)
  Demux/2 -> Subsystem1/1(q)
  Demux/1 -> Subsystem/1(q)
  Constant2/1 -> Demux2/1
  Matrix Multiply2/1 -> T_finger_tip/1
  Matrix Multiply1/1 -> Matrix Multiply2/1
  Subsystem3/1(Out1) -> Matrix Multiply2/2
  Subsystem2/1(Out1) -> Matrix Multiply1/2
  Matrix Multiply/1 -> Matrix Multiply1/1
  Subsystem1/1(Out1) -> Matrix Multiply/2
  Subsystem/1(Out1) -> Matrix Multiply3/2
  Constant/1 -> Demux1/1
  T_0f/1 -> Matrix Multiply3/1
  Matrix Multiply3/1 -> Matrix Multiply/1

== system Simulatore_COMAU_UBHAND_IV_manipolazione_interna/Work Space Control/Grasp Planner/SCOPES/Direct_kinematic2/Direct_kinematic_hand/Direct_kinematic_middle ==
  Joints_reference : Inport  PortDimensions=3
  T_0f : Inport  Port=2
  Constant : Constant  Value=[alpha_f(3,1) alpha_f(3,2) alpha_f(3,3) alpha_f(3,4)]
  Constant2 : Constant  Value=[a_f(3,1) a_f(3,2) a_f(3,3) a_f(3,4)]
  Degrees to Radians : simulink_extras/Transformations/Degrees to Radians
  Demux : Demux
  Demux1 : Demux
  Demux2 : Demux
  Demux3 : Demux  Outputs=3
  Display3 : Display
  Gain : Gain  Gain=0.7
  Matrix Multiply{,1..3} (4 alike) : Product  Multiplication=Matrix(*)
  Subsystem : Subsystem
  Subsystem1 : Subsystem
  Subsystem2 : Subsystem
  Subsystem3 : Subsystem
  Vector Concatenate : Concatenate  Mode=Vector
  T_finger_tip : Outport
  wires:
  Constant/1 -> Demux1/1
  Subsystem/1(Out1) -> Matrix Multiply3/2
  Subsystem1/1(Out1) -> Matrix Multiply/2
  Matrix Multiply/1 -> Matrix Multiply1/1
  Subsystem2/1(Out1) -> Matrix Multiply1/2
  Subsystem3/1(Out1) -> Matrix Multiply2/2
  Matrix Multiply1/1 -> Matrix Multiply2/1
  Matrix Multiply2/1 -> T_finger_tip/1
  Constant2/1 -> Demux2/1
  Demux/1 -> Subsystem/1(q)
  Demux/2 -> Subsystem1/1(q)
  Demux/3 -> Subsystem2/1(q)
  Demux/4 -> Subsystem3/1(q)
  Demux2/1 -> Subsystem/3(a)
  Demux2/2 -> Subsystem1/3(a)
  Demux2/3 -> Subsystem2/3(a)
  Demux2/4 -> Subsystem3/3(a)
  Demux1/1 -> Subsystem/2(alpha)
  Demux1/2 -> Subsystem1/2(alpha)
  Demux1/3 -> Subsystem2/2(alpha)
  Demux1/4 -> Subsystem3/2(alpha)
  Joints_reference/1 -> Demux3/1, Vector Concatenate/1
  Demux3/3 -> Gain/1
  Gain/1 -> Vector Concatenate/2
  Vector Concatenate/1 -> Degrees to Radians/1, Display3/1
  Degrees to Radians/1 -> Demux/1
  T_0f/1 -> Matrix Multiply3/1
  Matrix Multiply3/1 -> Matrix Multiply/1

== system Simulatore_COMAU_UBHAND_IV_manipolazione_interna/Work Space Control/Grasp Planner/SCOPES/Direct_kinematic2/Direct_kinematic_hand/Direct_kinematic_ring ==
  Joints_reference : Inport  PortDimensions=3
  T_0f : Inport  Port=2
  Constant : Constant  Value=[alpha_f(4,1) alpha_f(4,2) alpha_f(4,3) alpha_f(4,4)]
  Constant2 : Constant  Value=[a_f(4,1) a_f(4,2) a_f(4,3) a_f(4,4)]
  Degrees to Radians : simulink_extras/Transformations/Degrees to Radians
  Demux : Demux
  Demux1 : Demux
  Demux2 : Demux
  Demux3 : Demux  Outputs=3
  Display3 : Display
  Gain : Gain  Gain=0.7
  Matrix Multiply{,1..3} (4 alike) : Product  Multiplication=Matrix(*)
  Subsystem : Subsystem
  Subsystem1 : Subsystem
  Subsystem2 : Subsystem
  Subsystem3 : Subsystem
  Vector Concatenate : Concatenate  Mode=Vector
  T_finger_tip : Outport
  wires:
  Degrees to Radians/1 -> Demux/1
  Vector Concatenate/1 -> Display3/1, Degrees to Radians/1
  Gain/1 -> Vector Concatenate/2
  Demux3/3 -> Gain/1
  Joints_reference/1 -> Vector Concatenate/1, Demux3/1
  Demux1/4 -> Subsystem3/2(alpha)
  Demux1/3 -> Subsystem2/2(alpha)
  Demux1/2 -> Subsystem1/2(alpha)
  Demux1/1 -> Subsystem/2(alpha)
  Demux2/4 -> Subsystem3/3(a)
  Demux2/3 -> Subsystem2/3(a)
  Demux2/2 -> Subsystem1/3(a)
  Demux2/1 -> Subsystem/3(a)
  Demux/4 -> Subsystem3/1(q)
  Demux/3 -> Subsystem2/1(q)
  Demux/2 -> Subsystem1/1(q)
  Demux/1 -> Subsystem/1(q)
  Constant2/1 -> Demux2/1
  Matrix Multiply2/1 -> T_finger_tip/1
  Matrix Multiply1/1 -> Matrix Multiply2/1
  Subsystem3/1(Out1) -> Matrix Multiply2/2
  Subsystem2/1(Out1) -> Matrix Multiply1/2
  Matrix Multiply/1 -> Matrix Multiply1/1
  Subsystem1/1(Out1) -> Matrix Multiply/2
  Subsystem/1(Out1) -> Matrix Multiply3/2
  Constant/1 -> Demux1/1
  T_0f/1 -> Matrix Multiply3/1
  Matrix Multiply3/1 -> Matrix Multiply/1

== system Simulatore_COMAU_UBHAND_IV_manipolazione_interna/Work Space Control/Grasp Planner/SCOPES/Direct_kinematic2/Direct_kinematic_hand/Direct_kinematic_thumb ==
  Joints_reference : Inport  PortDimensions=3
  T_0f : Inport  Port=2
  Add : Sum
  Add1 : Sum  Inputs=-+
  Add2 : Sum
  Add3 : Sum
  Constant : Constant  Value=[alpha_f(1,1) alpha_f(1,2) alpha_f(1,3) alpha_f(1,4)]
  Constant1 : Constant  Value=teta_f(1,1)
  Constant2 : Constant  Value=[a_f(1,1) a_f(1,2) a_f(1,3) a_f(1,4)]
  Constant3 : Constant  Value=teta_f(1,2)
  Constant4 : Constant  Value=teta_f(1,3)
  Constant5 : Constant  Value=teta_f(1,4)
  Degrees to Radians : simulink_extras/Transformations/Degrees to Radians
  Demux : Demux
  Demux1 : Demux
  Demux2 : Demux
  Demux3 : Demux  Outputs=3
  Display3 : Display
  Gain : Gain  Gain=0.8
  Matrix Multiply{,1..3} (4 alike) : Product  Multiplication=Matrix(*)
  Subsystem : Subsystem
  Subsystem1 : Subsystem
  Subsystem2 : Subsystem
  Subsystem3 : Subsystem
  Vector Concatenate : Concatenate  Mode=Vector
  T_finger_tip : Outport
  T_thumb_base : Outport  Port=2
  wires:
  Constant/1 -> Demux1/1
  Subsystem/1(Out1) -> Matrix Multiply3/2
  Subsystem1/1(Out1) -> Matrix Multiply/2
  Matrix Multiply/1 -> Matrix Multiply1/1
  Subsystem2/1(Out1) -> Matrix Multiply1/2
  Subsystem3/1(Out1) -> Matrix Multiply2/2
  Matrix Multiply1/1 -> Matrix Multiply2/1
  Matrix Multiply2/1 -> T_finger_tip/1, T_thumb_base/1
  Constant2/1 -> Demux2/1
  Demux2/1 -> Subsystem/3(a)
  Demux2/2 -> Subsystem1/3(a)
  Demux2/3 -> Subsystem2/3(a)
  Demux2/4 -> Subsystem3/3(a)
  Demux1/1 -> Subsystem/2(alpha)
  Demux1/2 -> Subsystem1/2(alpha)
  Demux1/3 -> Subsystem2/2(alpha)
  Demux1/4 -> Subsystem3/2(alpha)
  Joints_reference/1 -> Demux3/1, Vector Concatenate/1
  Demux3/3 -> Gain/1
  Gain/1 -> Vector Concatenate/2
  Vector Concatenate/1 -> Degrees to Radians/1, Display3/1
  Degrees to Radians/1 -> Demux/1
  T_0f/1 -> Matrix Multiply3/1
  Matrix Multiply3/1 -> Matrix Multiply/1
  Constant1/1 -> Add/2
  Demux/1 -> Add/1
  Demux/2 -> Add1/1
  Demux/3 -> Add2/1
  Demux/4 -> Add3/1
  Add3/1 -> Subsystem3/1(q)
  Add2/1 -> Subsystem2/1(q)
  Add1/1 -> Subsystem1/1(q)
  Add/1 -> Subsystem/1(q)
  Constant3/1 -> Add1/2
  Constant4/1 -> Add2/2
  Constant5/1 -> Add3/2

== system Simulatore_COMAU_UBHAND_IV_manipolazione_interna/Work Space Control/Grasp Planner/SCOPES/Direct_kinematic2/Direct_kinematic_wrist/T_0_8 ==
  wrist_angles[1..2] : Inport
  T_0HAND : Inport  Port=2
  Add : Sum
  Add1 : Sum
  Constant : Constant  Value=[alpha_w(1) alpha_w(2)]
  Constant1 : Constant  Value=0
  Constant2 : Constant  Value=[a_w(1) a_w(2)]
  Constant3 : Constant  Value=0
  Degrees to Radians : simulink_extras/Transformations/Degrees to Radians
  Demux : Demux  Outputs=2
  Demux1 : Demux  Outputs=2
  Demux2 : Demux  Outputs=2
  Display3 : Display
  Matrix Multiply : Product  Multiplication=Matrix(*)
  Matrix Multiply1 : Product  Multiplication=Matrix(*)
  Matrix Multiply2 : Product  Multiplication=Matrix(*)
  Subsystem : Subsystem
  Subsystem1 : Subsystem
  wrist_reference_ : Subsystem
  T_08 : Outport
  wires:
  wrist_angles[1..2]/1 -> Display3/1, Degrees to Radians/1
  Degrees to Radians/1 -> Demux/1
  Demux1/2 -> Subsystem1/2(alpha)
  Demux1/1 -> Subsystem/2(alpha)
  Demux2/2 -> Subsystem1/3(a)
  Demux2/1 -> Subsystem/3(a)
  Constant2/1 -> Demux2/1
  Matrix Multiply/1 -> T_08/1
  Subsystem1/1(Out1) -> Matrix Multiply/2
  Constant/1 -> Demux1/1
  Subsystem/1(Out1) -> Matrix Multiply1/2
  Matrix Multiply1/1 -> Matrix Multiply/1
  Constant1/1 -> Add/2
  Demux/1 -> Add/1
  Add/1 -> Subsystem/1(q)
  Constant3/1 -> Add1/2
  Demux/2 -> Add1/1
  Add1/1 -> Subsystem1/1(q)
  T_0HAND/1 -> Matrix Multiply2/1
  wrist_reference_/1(T0_W) -> Matrix Multiply2/2
  Matrix Multiply2/1 -> Matrix Multiply1/1

== system Simulatore_COMAU_UBHAND_IV_manipolazione_interna/Work Space Control/Grasp Planner/box/punti di contatto box/object rotation matrix/R ==
  alpha : Inport
  beta : Inport  Port=2
  gamma : Inport  Port=3
  Matrix Multiply3 : Product  Multiplication=Matrix(*)
  Matrix Multiply4 : Product  Multiplication=Matrix(*)
  rot_x : Subsystem
  rot_y : Subsystem
  rot_z : Subsystem
  R : Outport
  wires:
  gamma/1 -> rot_z/1(gamma)
  beta/1 -> rot_y/1(beta)
  alpha/1 -> rot_x/1(alpha)
  rot_x/1(rotx) -> Matrix Multiply3/1
  rot_y/1(roty) -> Matrix Multiply3/2
  rot_z/1(rotz) -> Matrix Multiply4/2
  Matrix Multiply3/1 -> Matrix Multiply4/1
  Matrix Multiply4/1 -> R/1

== system Simulatore_COMAU_UBHAND_IV_manipolazione_interna/Work Space Control/Grasp Planner/box/punti di contatto box/object rotation matrix/R_01 ==
  alpha : Inport
  beta : Inport  Port=2
  gamma : Inport  Port=3
  Matrix Multiply3 : Product  Multiplication=Matrix(*)
  Matrix Multiply4 : Product  Multiplication=Matrix(*)
  rot_x : Subsystem
  rot_y : Subsystem
  rot_z : Subsystem
  R_01 : Outport
  wires:
  gamma/1 -> rot_z/1(gamma)
  beta/1 -> rot_y/1(beta)
  alpha/1 -> rot_x/1(alpha)
  rot_x/1(rotx) -> Matrix Multiply3/1
  rot_y/1(roty) -> Matrix Multiply3/2
  rot_z/1(rotz) -> Matrix Multiply4/2
  Matrix Multiply3/1 -> Matrix Multiply4/1
  Matrix Multiply4/1 -> R_01/1

== system Simulatore_COMAU_UBHAND_IV_manipolazione_interna/Work Space Control/Grasp Planner/box/punti di contatto box/object rotation matrix/R_56 ==
  alpha : Inport
  beta : Inport  Port=2
  gamma : Inport  Port=3
  Matrix Multiply3 : Product  Multiplication=Matrix(*)
  Matrix Multiply4 : Product  Multiplication=Matrix(*)
  rot_x : Subsystem
  rot_y : Subsystem
  rot_z : Subsystem
  R56 : Outport
  wires:
  Matrix Multiply4/1 -> R56/1
  Matrix Multiply3/1 -> Matrix Multiply4/1
  rot_z/1(rotz) -> Matrix Multiply4/2
  rot_y/1(roty) -> Matrix Multiply3/2
  rot_x/1(rotx) -> Matrix Multiply3/1
  alpha/1 -> rot_x/1(alpha)
  beta/1 -> rot_y/1(beta)
  gamma/1 -> rot_z/1(gamma)

== system Simulatore_COMAU_UBHAND_IV_manipolazione_interna/Work Space Control/Grasp Planner/box/punti di contatto box/object rotation matrix/definizione_T_0_object_tip1 ==
  box_pos : Inport
  R_01 : Inport  Port=2
  1pc : Inport  Port=3
  2pr : Inport  Port=4
  3pc : Inport  Port=5
  R : Inport  Port=6
  1pc1 : InportShadow  Port=3
  Display{,1..4} (5 alike) : Display
  Matrix Multiply{1..6,8..9} (8 alike) : Product  Multiplication=Matrix(*)
  Multiport Switch1 : MultiPortSwitch  Inputs=2  zeroidx=off
  R_1 : Constant  Value=eye(3)
  R_12 : Constant  Value=eye(3)
  Sum1 : Sum
  Sum3 : Sum  Inputs=+-
  Sum4 : Sum
  Sum5 : Sum
  0pc : Outport
  0pr : Outport  Port=2
  0pc' : Outport  Port=3
  R1 : Outport  Port=4
  R_05 : Outport  Port=5
  tag scope: reference_point_box=global
  wires:
  R_01/1 -> Matrix Multiply9/2, Matrix Multiply1/1, Matrix Multiply2/1, Matrix Multiply3/1, Multiport Switch1/2
  1pc/1 -> Matrix Multiply1/2
  box_pos/1 -> Sum3/1
  Matrix Multiply1/1 -> Sum3/2
  Sum3/1 -> 0pc/1, Sum4/1
  R_12/1 -> Matrix Multiply2/2
  Matrix Multiply2/1 -> Matrix Multiply5/1
  2pr/1 -> Matrix Multiply3/2
  Matrix Multiply3/1 -> Sum4/2, Display3/1
  Sum4/1 -> 0pr/1, Sum5/1, Display2/1
  R_1/1 -> Matrix Multiply5/2
  Sum5/1 -> Display/1, 0pc'/1, Sum1/1
  Matrix Multiply8/1 -> Display1/1, Sum5/2
  Matrix Multiply9/1 -> R_05/1, Multiport Switch1/3
  R/1 -> Matrix Multiply8/1, Matrix Multiply9/1, R1/1
  3pc/1 -> Matrix Multiply6/2
  Matrix Multiply6/1 -> Matrix Multiply8/2, Display4/1
  Sum1/1 -> [reference_point_box]
  Matrix Multiply4/1 -> Sum1/2
  1pc1/1 -> Matrix Multiply4/2
  Matrix Multiply5/1 -> Matrix Multiply6/1
  [orientation_mode_box] -> Multiport Switch1/1
  Multiport Switch1/1 -> Matrix Multiply4/1

== system Simulatore_COMAU_UBHAND_IV_manipolazione_interna/Work Space Control/Grasp Planner/box/punti di contatto box/punti di contatto sfera/Subsystem ==
  dimens1/2/side : Inport
  T_object : Inport  Port=2
  Constant : Constant
  Demux : Demux  Outputs=3
  Demux2 : Demux  Outputs=3
  Demux3 : Demux  Outputs=3
  Display : Display
  Display1 : Display
  Gain : Gain  Gain=-1
  Gain1 : Gain  Gain=-1
  Gain2 : Gain  Gain=-1
  Gain3 : Gain  Gain=1/2
  Matrix Multiply : Product  Multiplication=Matrix(*)
  Multiport Switch : MultiPortSwitch  Inputs=6  zeroidx=off
  Mux : Mux  Inputs=2
  Mux{1..6} (6 alike) : Mux  Inputs=3
  R_0EE1 : dspmtrx3/Submatrix  RowSpan=Range of rows  RowStartMode=Index  RowStartIndex=1  RowEndMode=Index  RowEndIndex=3  ColSpan=One column  ColStartMode=Index  ColStartIndex=4  ColEndMode=Index  ColEndIndex=4
  R_0EE2 : dspmtrx3/Submatrix  RowSpan=Range of rows  RowStartMode=Index  RowStartIndex=1  RowEndMode=Index  RowEndIndex=3  ColSpan=Range of columns  ColStartMode=Index  ColStartIndex=1  ColEndMode=Index  ColEndIndex=3
  Sum : Sum  Inputs=|++
  Sum3 : Sum
  Sum4 : Sum  Inputs=|++
  Sum5 : Sum  Inputs=|++
  dimens1/2/side1 : InportShadow  Port=1
  xyz 0 : Outport
  wires:
  [box_size] -> Gain3/1
  [radius_spheres] -> Sum/1
  Mux/1 -> xyz 0/1
  Sum3/1 -> Mux/1
  dimens1/2/side/1 -> Demux2/1
  Constant/1 -> Mux/2
  Demux2/3 -> Multiport Switch/1
  dimens1/2/side1/1 -> Demux3/1
  Sum/1 -> Mux1/1, Gain/1, Display/1
  Gain/1 -> Mux2/1
  [radius_spheres] -> Sum4/1
  Demux/2 -> Sum4/2
  Sum4/1 -> Gain1/1, Mux5/3
  Sum5/1 -> Gain2/1, Mux3/2
  [radius_spheres] -> Sum5/2
  Demux3/2 -> [dim2]
  Demux3/1 -> [dim1]
  [dim2] -> Mux1/3
  [dim1] -> Mux1/2
  [dim2] -> Mux2/3
  [dim1] -> Mux2/2
  [dim1] -> Mux5/1
  [dim1] -> Mux6/1
  [dim1] -> Mux3/1
  [dim1] -> Mux4/1
  Mux1/1 -> Multiport Switch/2
  Mux2/1 -> Multiport Switch/3
  Mux5/1 -> Multiport Switch/4
  Mux6/1 -> Multiport Switch/5
  Mux3/1 -> Multiport Switch/6
  Mux4/1 -> Multiport Switch/7
  Gain2/1 -> Mux4/2
  [dim2] -> Mux4/3
  [dim2] -> Mux3/3
  Demux/3 -> Sum/2
  Demux/1 -> Sum5/1
  Gain3/1 -> Demux/1, Display1/1
  [dim2] -> Mux6/2
  Gain1/1 -> Mux6/3
  [dim2] -> Mux5/2
  Matrix Multiply/1 -> Sum3/1
  Multiport Switch/1 -> Matrix Multiply/2
  T_object/1 -> R_0EE2/1, R_0EE1/1
  R_0EE2/1 -> Matrix Multiply/1
  R_0EE1/1 -> Sum3/2

== system Simulatore_COMAU_UBHAND_IV_manipolazione_interna/Work Space Control/Grasp Planner/box/punti di contatto box/punti di contatto sfera/Subsystem1 ==
  dimens1/2/side : Inport
  T_object : Inport  Port=2
  Constant : Constant
  Demux : Demux  Outputs=3
  Demux2 : Demux  Outputs=3
  Demux3 : Demux  Outputs=3
  Display : Display
  Display1 : Display
  Gain : Gain  Gain=-1
  Gain1 : Gain  Gain=-1
  Gain2 : Gain  Gain=-1
  Gain3 : Gain  Gain=1/2
  Matrix Multiply : Product  Multiplication=Matrix(*)
  Multiport Switch : MultiPortSwitch  Inputs=6  zeroidx=off
  Mux : Mux  Inputs=2
  Mux{1..6} (6 alike) : Mux  Inputs=3
  R_0EE1 : dspmtrx3/Submatrix  RowSpan=Range of rows  RowStartMode=Index  RowStartIndex=1  RowEndMode=Index  RowEndIndex=3  ColSpan=One column  ColStartMode=Index  ColStartIndex=4  ColEndMode=Index  ColEndIndex=4
  R_0EE2 : dspmtrx3/Submatrix  RowSpan=Range of rows  RowStartMode=Index  RowStartIndex=1  RowEndMode=Index  RowEndIndex=3  ColSpan=Range of columns  ColStartMode=Index  ColStartIndex=1  ColEndMode=Index  ColEndIndex=3
  Sum : Sum  Inputs=|++
  Sum3 : Sum
  Sum4 : Sum  Inputs=|++
  Sum5 : Sum  Inputs=|++
  dimens1/2/side1 : InportShadow  Port=1
  xyz 0 : Outport
  wires:
  R_0EE1/1 -> Sum3/2
  R_0EE2/1 -> Matrix Multiply/1
  T_object/1 -> R_0EE1/1, R_0EE2/1
  Multiport Switch/1 -> Matrix Multiply/2
  Matrix Multiply/1 -> Sum3/1
  [dim2] -> Mux5/2
  Gain1/1 -> Mux6/3
  [dim2] -> Mux6/2
  Gain3/1 -> Display1/1, Demux/1
  Demux/1 -> Sum5/1
  Demux/3 -> Sum/2
  [dim2] -> Mux3/3
  [dim2] -> Mux4/3
  Gain2/1 -> Mux4/2
  Mux4/1 -> Multiport Switch/7
  Mux3/1 -> Multiport Switch/6
  Mux6/1 -> Multiport Switch/5
  Mux5/1 -> Multiport Switch/4
  Mux2/1 -> Multiport Switch/3
  Mux1/1 -> Multiport Switch/2
  [dim1] -> Mux4/1
  [dim1] -> Mux3/1
  [dim1] -> Mux6/1
  [dim1] -> Mux5/1
  [dim1] -> Mux2/2
  [dim2] -> Mux2/3
  [dim1] -> Mux1/2
  [dim2] -> Mux1/3
  Demux3/1 -> [dim1]
  Demux3/2 -> [dim2]
  [radius_spheres] -> Sum5/2
  Sum5/1 -> Mux3/2, Gain2/1
  Sum4/1 -> Mux5/3, Gain1/1
  Demux/2 -> Sum4/2
  [radius_spheres] -> Sum4/1
  Gain/1 -> Mux2/1
  Sum/1 -> Display/1, Gain/1, Mux1/1
  dimens1/2/side1/1 -> Demux3/1
  Demux2/3 -> Multiport Switch/1
  Constant/1 -> Mux/2
  dimens1/2/side/1 -> Demux2/1
  Sum3/1 -> Mux/1
  Mux/1 -> xyz 0/1
  [radius_spheres] -> Sum/1
  [box_size] -> Gain3/1

== system Simulatore_COMAU_UBHAND_IV_manipolazione_interna/Work Space Control/Grasp Planner/box/punti di contatto box/punti di contatto sfera/Subsystem2 ==
  dimens1/2/side : Inport
  T_object : Inport  Port=2
  Constant : Constant
  Demux : Demux  Outputs=3
  Demux2 : Demux  Outputs=3
  Demux3 : Demux  Outputs=3
  Display : Display
  Display1 : Display
  Gain : Gain  Gain=-1
  Gain1 : Gain  Gain=-1
  Gain2 : Gain  Gain=-1
  Gain3 : Gain  Gain=1/2
  Matrix Multiply : Product  Multiplication=Matrix(*)
  Multiport Switch : MultiPortSwitch  Inputs=6  zeroidx=off
  Mux : Mux  Inputs=2
  Mux{1..6} (6 alike) : Mux  Inputs=3
  R_0EE1 : dspmtrx3/Submatrix  RowSpan=Range of rows  RowStartMode=Index  RowStartIndex=1  RowEndMode=Index  RowEndIndex=3  ColSpan=One column  ColStartMode=Index  ColStartIndex=4  ColEndMode=Index  ColEndIndex=4
  R_0EE2 : dspmtrx3/Submatrix  RowSpan=Range of rows  RowStartMode=Index  RowStartIndex=1  RowEndMode=Index  RowEndIndex=3  ColSpan=Range of columns  ColStartMode=Index  ColStartIndex=1  ColEndMode=Index  ColEndIndex=3
  Sum : Sum  Inputs=|++
  Sum3 : Sum
  Sum4 : Sum  Inputs=|++
  Sum5 : Sum  Inputs=|++
  dimens1/2/side1 : InportShadow  Port=1
  xyz 0 : Outport
  wires:
  [box_size] -> Gain3/1
  [radius_spheres] -> Sum/1
  Mux/1 -> xyz 0/1
  Sum3/1 -> Mux/1
  dimens1/2/side/1 -> Demux2/1
  Constant/1 -> Mux/2
  Demux2/3 -> Multiport Switch/1
  dimens1/2/side1/1 -> Demux3/1
  Sum/1 -> Mux1/1, Gain/1, Display/1
  Gain/1 -> Mux2/1
  [radius_spheres] -> Sum4/1
  Demux/2 -> Sum4/2
  Sum4/1 -> Gain1/1, Mux5/3
  Sum5/1 -> Gain2/1, Mux3/2
  [radius_spheres] -> Sum5/2
  Demux3/2 -> [dim2]
  Demux3/1 -> [dim1]
  [dim2] -> Mux1/3
  [dim1] -> Mux1/2
  [dim2] -> Mux2/3
  [dim1] -> Mux2/2
  [dim1] -> Mux5/1
  [dim1] -> Mux6/1
  [dim1] -> Mux3/1
  [dim1] -> Mux4/1
  Mux1/1 -> Multiport Switch/2
  Mux2/1 -> Multiport Switch/3
  Mux5/1 -> Multiport Switch/4
  Mux6/1 -> Multiport Switch/5
  Mux3/1 -> Multiport Switch/6
  Mux4/1 -> Multiport Switch/7
  Gain2/1 -> Mux4/2
  [dim2] -> Mux4/3
  [dim2] -> Mux3/3
  Demux/3 -> Sum/2
  Demux/1 -> Sum5/1
  Gain3/1 -> Demux/1, Display1/1
  [dim2] -> Mux6/2
  Gain1/1 -> Mux6/3
  [dim2] -> Mux5/2
  Matrix Multiply/1 -> Sum3/1
  Multiport Switch/1 -> Matrix Multiply/2
  T_object/1 -> R_0EE2/1, R_0EE1/1
  R_0EE2/1 -> Matrix Multiply/1
  R_0EE1/1 -> Sum3/2
